simulink model "benchmark"  (Simulink 7.9)
settings: Solver=ode45  StartTime=0.0  StopTime=1000  RelTol=1e-3
undefined symbols (not defined in the file or in its folder): sigbuilder_block
libraries: libturbines x10, simulink x1
(part 3 of 3)

== matlab function Wind Field/Wake9/Wake deficit and centerline ==
  data: uy input double; CT input double; p parameter double; w_c output double; a output double
function [w_c,a]=wake(uy,CT,p)
    % Todo: Change length(uy)*grid_size to farm size?
    %       Change such that parm is passed directly

    w_c=zeros(length(p.farm.x),1);
    a=ones(length(p.farm.x),1);
    
    %Find wake center and deficit for all other turbines
    for i=1:length(p.farm.x)
        if(p.farm.x(i)-p.wt.x>0) %Is turbine P downwind?
            w_c(i)=max(min(wakecenter(uy,p.farm.x(i),p),p.grid.ysize),1);
            a(i)=deficit(CT,p.farm.x(i),p);
        else
            w_c(i)=p.wt.y;
            a(i)=1;
        end
    end
end


function wc=wakecenter(uy,px,p)
    
    %computer number of grid points to px
    it=ceil((px-p.wt.x)/p.grid.size);
    
    wc=p.wt.y; %wake center starts at turbine position
    
    for i=1:it %Compute the meandering for each grid point
        uysum=0;
        %compute the grid number for low meandering edge
        m_min=max(floor((wc-p.wt.meand.r(i))/p.grid.size)+1,1); 
        %compute the grid number for the high meandering edge
        m_max=min(ceil((wc+p.wt.meand.r(i))/p.grid.size)+1,length(uy)); 
        
        %average transversal velocity over the wake area
        for j=m_min:m_max
            uysum=uysum+uy(j);
        end
        uyavg=uysum/(m_max-m_min+1);
        
        % wake center filtered
        % Should be calculated from mean air speed vs uyavg and lowpass filtered
        % Redo filter computation
        % yeff=ts*uyavg=grid.size/uxmean*uyavg
        % wc=wc+alpha*uyavg
        
        %Uncomment to reproduce bug
        %wc=p.wt.y;
        
        %Uncomment to reproduce meandering limits
        %wc=min(max(wc,p.wt.y-150),p.wt.y+150);
        
        %Notice here ts such that wake moves with uyavg speed
        wc=wc+p.ts*uyavg;
    end
end

function a=deficit(CT,px,p)
    %wake deficit at px-wtx distance from turbine
    a=1-.5*CT/(1+(px-p.wt.x)/(4*p.wt.rotor.r));
end

== matlab function Wind Field/Wind Speed9/Wind speed ==
  data: ux input double; w_c input double; a input double; p parameter double; uavg output double; upoint output double
function [uavg,upoint]=windspeed(ux,w_c,a,p)
% ux    is the wind vector at the x position of the turbine
% w_c   is the vector of wake centers from the other turbins at the x position of the turbine
% a     is the vector of velocity deficits at this turbine from the other turbines
% P     is a structure containing parameters  
   
%Initialise variables
uxsum=0;
av=ones(p.grid.ysize,1);

%Merge the wake from all turbines upwind
for i=1:length(p.farm.x)
    if(p.farm.x(i)<p.wt.x) %only compute deficit effect from upstream turbines

        %compute the grid number for low meandering edge
        mn=floor((w_c(i)-p.wt.exprad(i))/p.grid.size)+1;
        m_min=max(mn,1); 
        %compute the grid number for the high meandering edge
        mx=floor((w_c(i)+p.wt.exprad(i))/p.grid.size)+1;
        m_max=min(mx,floor(p.grid.ysize/p.grid.size)+1);

        %Merge wakes
        for j=m_min:m_max
            av(j)=av(j)*a(i);
        end
    end
end
    


%Rotor grid points - Todo move to farm setup
%compute the grid number for low rotor edge
r_min=floor((p.wt.y-p.wt.rotor.r)/p.grid.size)+1; 
%compute the grid number for the high rotor edge
r_max=floor((p.wt.y+p.wt.rotor.r)/p.grid.size)+1;

%Sum the wake corrected x-velocities over the rotor dish area
for i=r_min:r_max
    uxsum=uxsum+ux(i)*av(i);
end

%Compute the average
uavg=uxsum/(r_max-r_min+1);

%Extract the wake corrected velocity at the nacelle
upoint=ux(floor(p.wt.y/p.grid.size)+1)*av(floor(p.wt.y/p.grid.size)+1);
    
end

== matlab function Wind Field/Wake10/Wake deficit and centerline ==
  data: uy input double; CT input double; p parameter double; w_c output double; a output double
function [w_c,a]=wake(uy,CT,p)
    % Todo: Change length(uy)*grid_size to farm size?
    %       Change such that parm is passed directly

    w_c=zeros(length(p.farm.x),1);
    a=ones(length(p.farm.x),1);
    
    %Find wake center and deficit for all other turbines
    for i=1:length(p.farm.x)
        if(p.farm.x(i)-p.wt.x>0) %Is turbine P downwind?
            w_c(i)=max(min(wakecenter(uy,p.farm.x(i),p),p.grid.ysize),1);
            a(i)=deficit(CT,p.farm.x(i),p);
        else
            w_c(i)=p.wt.y;
            a(i)=1;
        end
    end
end


function wc=wakecenter(uy,px,p)
    
    %computer number of grid points to px
    it=ceil((px-p.wt.x)/p.grid.size);
    
    wc=p.wt.y; %wake center starts at turbine position
    
    for i=1:it %Compute the meandering for each grid point
        uysum=0;
        %compute the grid number for low meandering edge
        m_min=max(floor((wc-p.wt.meand.r(i))/p.grid.size)+1,1); 
        %compute the grid number for the high meandering edge
        m_max=min(ceil((wc+p.wt.meand.r(i))/p.grid.size)+1,length(uy)); 
        
        %average transversal velocity over the wake area
        for j=m_min:m_max
            uysum=uysum+uy(j);
        end
        uyavg=uysum/(m_max-m_min+1);
        
        % wake center filtered
        % Should be calculated from mean air speed vs uyavg and lowpass filtered
        % Redo filter computation
        % yeff=ts*uyavg=grid.size/uxmean*uyavg
        % wc=wc+alpha*uyavg
        
        %Uncomment to reproduce bug
        %wc=p.wt.y;
        
        %Uncomment to reproduce meandering limits
        %wc=min(max(wc,p.wt.y-150),p.wt.y+150);
        
        %Notice here ts such that wake moves with uyavg speed
        wc=wc+p.ts*uyavg;
    end
end

function a=deficit(CT,px,p)
    %wake deficit at px-wtx distance from turbine
    a=1-.5*CT/(1+(px-p.wt.x)/(4*p.wt.rotor.r));
end

== matlab function Wind Field/Wind Speed10/Wind speed ==
  data: ux input double; w_c input double; a input double; p parameter double; uavg output double; upoint output double
function [uavg,upoint]=windspeed(ux,w_c,a,p)
% ux    is the wind vector at the x position of the turbine
% w_c   is the vector of wake centers from the other turbins at the x position of the turbine
% a     is the vector of velocity deficits at this turbine from the other turbines
% P     is a structure containing parameters  
   
%Initialise variables
uxsum=0;
av=ones(p.grid.ysize,1);

%Merge the wake from all turbines upwind
for i=1:length(p.farm.x)
    if(p.farm.x(i)<p.wt.x) %only compute deficit effect from upstream turbines

        %compute the grid number for low meandering edge
        mn=floor((w_c(i)-p.wt.exprad(i))/p.grid.size)+1;
        m_min=max(mn,1); 
        %compute the grid number for the high meandering edge
        mx=floor((w_c(i)+p.wt.exprad(i))/p.grid.size)+1;
        m_max=min(mx,floor(p.grid.ysize/p.grid.size)+1);

        %Merge wakes
        for j=m_min:m_max
            av(j)=av(j)*a(i);
        end
    end
end
    


%Rotor grid points - Todo move to farm setup
%compute the grid number for low rotor edge
r_min=floor((p.wt.y-p.wt.rotor.r)/p.grid.size)+1; 
%compute the grid number for the high rotor edge
r_max=floor((p.wt.y+p.wt.rotor.r)/p.grid.size)+1;

%Sum the wake corrected x-velocities over the rotor dish area
for i=r_min:r_max
    uxsum=uxsum+ux(i)*av(i);
end

%Compute the average
uavg=uxsum/(r_max-r_min+1);

%Extract the wake corrected velocity at the nacelle
upoint=ux(floor(p.wt.y/p.grid.size)+1)*av(floor(p.wt.y/p.grid.size)+1);
    
end

== matlab function Farm Control/control ==
  data: v_nac input double; P_demand input double; parm parameter double; P_ref output double; P_avail output double
function [P_ref,P_avail]  = Farm_Controller(v_nac,P_demand,parm)
%
% Farm_Controller estimates the available power at the farm and distributes the 
% power references proportional to the available power at each wind turbine.
%
% [P_ref,P_avail_total]  = Farm_Controller(v_nac,P_demand)
%
% vn : the wind speed at wind turbine number n.
%
% P_demand : The demanded power by the operator.
%
% P_ref : The power reference for the wind turbines.
%
% P_avail : Available power at the farm level
%
%
% Version 1: 2009-04-10 sms
% Version 2: 2009-12-04 grunnet
% By : S. Mohsen N. Soltani & Jacob D. Grunnet
% Aalborg University, Dept. of Electronic Systems, Section of Automation
% and Control
% E-mail: sms@es.aau.dk
% E-mail: grunnet@es.aau.dk

rho=parm.rho; %air dencity
R=parm.radius; %rotor radius
rated=parm.rated; %Rated power
N=parm.N; %Number of turbines
Cp=parm.Cp; % Max cp of the turbines

P_a=zeros(N,1);
P_ref=zeros(N,1);

% Compute available power at each turbine
for i=1:N
    %P_a=1/2*pi*r^2*Cp*v^3
    P_a(i)=min(rated(i),(pi/2)*rho*R(i)^2*v_nac(i)^3*Cp(i));
end

%Compute total available power
P_avail=sum(P_a);

%Distribute power according to availibility
for i=1:N
    P_ref(i)=max(0,min(rated(i),P_demand*P_a(i)/P_avail));
end

== matlab function Network Operator/Network Operator ==
  data: P_avail input double; settings input double; f input double; P_demand output double; P_demand0 input double; parm parameter double
function P_demand = TSO(P_avail,settings,f,P_demand0,parm)
%
% TSO provides the demanded power to the wind farm controller.
%
% P_avail : Aavailable power at the farm level.
%
% mode : The operation mode.
%
% settings : The settings corresponding to the operational mode
%
% f : Frequency of the network
%
% P_deamnd0 : The last update of the deamded power
%
% Ts : Sample Time
%
% Time-stamp: <2009-04-10 sms>
% Version 1: 2009-04-10 sms 
% By : S. Mohsen N. Soltani
% Aalborg University, Dept. of Electronic Systems, Section of Automation
% and Control
% E-mail: sms@es.aau.dk
% 
 P=0;
 switch(parm.mode)
     case 1 %Absolute active power control
        P=settings(1);
%       % type='Full power';
     case 2 %Delta mode
        P=P_avail-settings(1);
%       % type='Delta mode';
     case 3 %Balance
        P=settings(1);
%       % type='Balance';
     case 4 %Rate limiter
        P=min(P_avail,settings(1)*parm.Ts+P_demand0);
%       % type='Rate limiter';
     case 5 %Frequency Control
        deadb=settings(1);
        contb=settings(2);
        maxp=settings(3);
        minp=settings(4);
        f=f-settings(5); % To provide Delta f
        if ( f<= deadb && f>=-deadb)
            P=0.5*(maxp+minp);
        elseif f>= contb
            P=minp;
        elseif f<= -contb
            P=maxp;
        elseif ( f>deadb && f<contb)
            P=0.5*(maxp+minp)-0.5*(maxp-minp)*(f-deadb)/(contb-deadb);
        elseif ( f<-deadb && f>-contb)
            P=0.5*(maxp+minp)-0.5*(maxp-minp)*(f+deadb)/(contb-deadb);
        end
%       % type='Frequency Control';
     otherwise %Unknown
        P=P_avail;
%       % type='Unknown Mode';
 end
%        

P_demand=max(0,P);
